simulink model "BaseEngineController_A02"  (Simulink 7.7)
settings: Solver=FixedStepDiscrete  StartTime=0.0  StopTime=10.0  RelTol=1e-3
PreLoadFcn:
  | try, eval([bdroot '_setup']); catch, eval('model_setup', ''); end
InitFcn:
  | try, eval([bdroot '_setup']); catch, eval('model_setup', ''); end
undefined symbols (not defined in the file or in its folder): ECU555, High, motohawk_build_version, motohawk_get_faults, motohawk_trunc_name, motohawk_vardec_config_group, motohawk_vardec_path, True
libraries: MotoHawk_lib x1145, AutoDoc_lib x786, motohawk_control_primitives_lib x114, motohawk_engine_lib x19, motohawk_annotations_lib x12, simulink x11, simulink_extras x1
(part 18 of 23)

== system BaseEngineController_A02/Foreground/Control/Controller/Run/AirFlow Manager/Power Limit Controller ==
  ETC Setpoint : Inport
  Sensors : Inport  Port=2
  Add : Sum
  Add1 : Sum  Inputs=+-
  Bus Selector1 : BusSelector  OutputSignals=TorqueCalcs.Power
  Compare To Zero : simulink/Logic and Bit Operations/Compare To Zero  SystemSampleTime=-1  relop===  LogicOutDataTypeMode=boolean  ZeroCross=off
  Constant1 : Constant  Value=100
  Constant2 : Constant  Value=100
  Documentation_Calibrations_Probes_Block : AutoDoc_lib/Documentation_Calibrations_Probes_Block
  Documentation_Left_Border : AutoDoc_lib/Documentation_Left_Border  layout=Portrait
  Documentation_Right_Border : AutoDoc_lib/Documentation_Right_Border  layout=Portrait
  Documentation_Title_Block : AutoDoc_lib/Documentation_Title_Block
  MinMax : MinMax  Inputs=2
  Power Limiter Setpoint Manager : Stateflow chart  TreatAsAtomicUnit=on
  Unit Delay : UnitDelay  SampleTime=-1
  Universal PID (Tunable & Scheduled) : Subsystem  AncestorBlock=motohawk_control_primitives_lib/Universal PID (Tunable & Scheduled)  mask(Universal PID (Tunable)){nam='PowerLimt', prefix='PowerLimt_', DCOffset=0, PGain=0, IGain=0, DGain=0, ErrorGain=-1, ITermLwrLim=-100, ITermUprLim=0, OutLwrLim=-100, OutUprLim=0, samp_time=-1, units='%', group='Diagnostics & Protection | Power Limit | Power Limit PID Config'}
      mask prompts: nam "Name"; DCOffset "DC Offset"; ErrorGain "Error Pre-scaler Gain"; ITermLwrLim "I-Term Lower Limit"; ITermUprLim "I-Term Upper Limit"; OutLwrLim "PID Output Lower Limit"; OutUprLim "PID Output Upper Limit"; samp_time "Sample Time"; group "MotoTune Group String"
      mask help:
      | This block wraps a 'Universal PID' block to provide gain scheduling and calibrations and probes for most parameters.
      |
      | If 'Sample Time' is -1, then extra code and RAM will be generated to dynamically calculate dt.
      mask init:
      | grp = [group ' | ' nam];
      mask Display:
      | patch([0 0 1 1], [0 0.075 0.075 0], [230/255 0/255 0/255])
      | patch([0 0 1 1], [0.025 0.05 0.05 0.025], [150/255 0/255 0/255])
      | patch([0 0 1 1], [0 0.025 0.025 0], [0/255 0/255 0/255])
      | 
      | disp([nam '\n(PID w/ Gain Schedules)']);
  motohawk_calibration : MotoHawk_lib/Calibration & Probing Blocks/motohawk_calibration  nam='PowerLimit1'  val=60  storage=Calibration  show_more=on  name_source=Use Parameter  data_type=Inherit from 'Default Value'  read_level=1  write_level=1  ovr_cals=on  view_as=Number  enum_struct={'Disabled', 'Enabled'}  vector_style=Wide Row  use_help_units=on  help='Power Limit setpoint corresponding to Level 1.'  units='kW'  use_header_enum=off  row_header_enum={}  col_header_enum={}  use_min_max=off  min_val=-inf  max_val=inf  use_precision=off  precision=''  gain=1  offset=0  exp=1  use_mototune_group=on  mototune_group='Diagnostics & Protection | Power Limit | Power Limit Level Config'
  motohawk_calibration1 : MotoHawk_lib/Calibration & Probing Blocks/motohawk_calibration  nam='PowerLimit2'  val=40  storage=Calibration  show_more=on  name_source=Use Parameter  data_type=Inherit from 'Default Value'  read_level=1  write_level=1  ovr_cals=on  view_as=Number  enum_struct={'Disabled', 'Enabled'}  vector_style=Wide Row  use_help_units=on  help='Power Limit setpoint corresponding to Level 2.'  units='kW'  use_header_enum=off  row_header_enum={}  col_header_enum={}  use_min_max=off  min_val=-inf  max_val=inf  use_precision=off  precision=''  gain=1  offset=0  exp=1  use_mototune_group=on  mototune_group='Diagnostics & Protection | Power Limit | Power Limit Level Config'
  motohawk_calibration2 : MotoHawk_lib/Calibration & Probing Blocks/motohawk_calibration  nam='PowerLimit3'  val=30  storage=Calibration  show_more=on  name_source=Use Parameter  data_type=Inherit from 'Default Value'  read_level=1  write_level=1  ovr_cals=on  view_as=Number  enum_struct={'Disabled', 'Enabled'}  vector_style=Wide Row  use_help_units=on  help='Power Limit setpoint corresponding to Level 3.'  units='kW'  use_header_enum=off  row_header_enum={}  col_header_enum={}  use_min_max=off  min_val=-inf  max_val=inf  use_precision=off  precision=''  gain=1  offset=0  exp=1  use_mototune_group=on  mototune_group='Diagnostics & Protection | Power Limit | Power Limit Level Config'
  motohawk_calibration3 : MotoHawk_lib/Calibration & Probing Blocks/motohawk_calibration  nam='PowerLimit4'  val=20  storage=Calibration  show_more=on  name_source=Use Parameter  data_type=Inherit from 'Default Value'  read_level=1  write_level=1  ovr_cals=on  view_as=Number  enum_struct={'Disabled', 'Enabled'}  vector_style=Wide Row  use_help_units=on  help='Power Limit setpoint corresponding to Level 4.'  units='kW'  use_header_enum=off  row_header_enum={}  col_header_enum={}  use_min_max=off  min_val=-inf  max_val=inf  use_precision=off  precision=''  gain=1  offset=0  exp=1  use_mototune_group=on  mototune_group='Diagnostics & Protection | Power Limit | Power Limit Level Config'
  motohawk_calibration4 : MotoHawk_lib/Calibration & Probing Blocks/motohawk_calibration  nam='EngMaxPower'  val=60  storage=Calibration  show_more=on  name_source=Use Parameter  data_type=Inherit from 'Default Value'  read_level=1  write_level=1  ovr_cals=on  view_as=Number  enum_struct={'Disabled', 'Enabled'}  vector_style=Wide Row  use_help_units=on  help='Maximum power level of engine.'  units='kW'  use_header_enum=off  row_header_enum={}  col_header_enum={}  use_min_max=off  min_val=-inf  max_val=inf  use_precision=off  precision=''  gain=1  offset=0  exp=1  use_mototune_group=on  mototune_group='Diagnostics & Protection | Power Limit | Power Limit Level Config'
  motohawk_fault_action : MotoHawk_lib/Fault Management Blocks/motohawk_fault_action  nam='Level1PowerLimit'  output_status=on  eval_nam=Level1PowerLimit
  motohawk_fault_action1 : MotoHawk_lib/Fault Management Blocks/motohawk_fault_action  nam='Level2PowerLimit'  output_status=on  eval_nam=Level2PowerLimit
  motohawk_fault_action2 : MotoHawk_lib/Fault Management Blocks/motohawk_fault_action  nam='Level3PowerLimit'  output_status=on  eval_nam=Level3PowerLimit
  motohawk_fault_action3 : MotoHawk_lib/Fault Management Blocks/motohawk_fault_action  nam='Level4PowerLimit'  output_status=on  eval_nam=Level4PowerLimit
  motohawk_probe : MotoHawk_lib/Calibration & Probing Blocks/motohawk_probe  nam='PowerLimitETCSetpoint'  show_more=on  name_source=Use Parameter  read_level=1  view_as=Number  enum_struct={'Disabled', 'Enabled'}  vector_style=Wide Row  use_help_units=off  help=''  units=''  use_header_enum=off  row_header_enum={}  col_header_enum={}  use_precision=off  precision=''  gain=1  offset=0  exp=1  use_mototune_group=on  mototune_group='Diagnostics & Protection | Power Limit'
  motohawk_probe1 : MotoHawk_lib/Calibration & Probing Blocks/motohawk_probe  nam='PowerLimitSetpoint'  show_more=on  name_source=Use Parameter  read_level=1  view_as=Number  enum_struct={'Disabled', 'Enabled'}  vector_style=Wide Row  use_help_units=off  help=''  units=''  use_header_enum=off  row_header_enum={}  col_header_enum={}  use_precision=off  precision=''  gain=1  offset=0  exp=1  use_mototune_group=on  mototune_group='Diagnostics & Protection | Power Limit'
  motohawk_probe2 : MotoHawk_lib/Calibration & Probing Blocks/motohawk_probe  nam='PowerLimitState'  show_more=on  name_source=Use Parameter  read_level=1  view_as=Number  enum_struct={'Disabled', 'Enabled'}  vector_style=Wide Row  use_help_units=off  help=''  units=''  use_header_enum=off  row_header_enum={}  col_header_enum={}  use_precision=off  precision=''  gain=1  offset=0  exp=1  use_mototune_group=on  mototune_group='Diagnostics & Protection | Power Limit'
  motohawk_probe3 : MotoHawk_lib/Calibration & Probing Blocks/motohawk_probe  nam='PowerLimitActual'  show_more=on  name_source=Use Parameter  read_level=1  view_as=Number  enum_struct={'Disabled', 'Enabled'}  vector_style=Wide Row  use_help_units=off  help=''  units=''  use_header_enum=off  row_header_enum={}  col_header_enum={}  use_precision=off  precision=''  gain=1  offset=0  exp=1  use_mototune_group=on  mototune_group='Diagnostics & Protection | Power Limit'
  PowerLimitETC : Outport
  wires:
  Constant2/1 -> Add1/2
  Compare To Zero/1 -> Universal PID (Tunable & Scheduled)/2(Reset All)
  Unit Delay/1 -> Power Limiter Setpoint Manager/11(PL_ETC)
  Power Limiter Setpoint Manager/1(State) -> Compare To Zero/1, motohawk_probe2/1
  motohawk_fault_action3/1 -> Power Limiter Setpoint Manager/10(Level4)
  motohawk_fault_action2/1 -> Power Limiter Setpoint Manager/9(Level3)
  motohawk_fault_action1/1 -> Power Limiter Setpoint Manager/8(Level2)
  motohawk_fault_action/1 -> Power Limiter Setpoint Manager/7(Level1)
  Power Limiter Setpoint Manager/2(Setpoint) -> motohawk_probe1/1, Universal PID (Tunable & Scheduled)/4(SetPoint)
  motohawk_calibration3/1 -> Power Limiter Setpoint Manager/6(Level4Setpoint)
  motohawk_calibration2/1 -> Power Limiter Setpoint Manager/5(Level3Setpoint)
  motohawk_calibration1/1 -> Power Limiter Setpoint Manager/4(Level2Setpoint)
  motohawk_calibration/1 -> Power Limiter Setpoint Manager/3(Level1Setpoint)
  motohawk_calibration4/1 -> Power Limiter Setpoint Manager/2(MaxEngPower)
  Bus Selector1/1 -> Universal PID (Tunable & Scheduled)/3(Actual), motohawk_probe3/1  "<Power>"
  Sensors/1 -> Bus Selector1/1
  Constant1/1 -> Add/1
  Add/1 -> Unit Delay/1, motohawk_probe/1, MinMax/2
  Universal PID (Tunable & Scheduled)/1(PIDOut) -> Add/2
  ETC Setpoint/1 -> Add1/1, Power Limiter Setpoint Manager/1(ETC), MinMax/1
  Add1/1 -> Universal PID (Tunable & Scheduled)/1(ITerm IC)
  MinMax/1 -> PowerLimitETC/1

== system BaseEngineController_A02/Foreground/Control/Controller/Run/AirFlow Manager/ShutDownTasksComplete Reset ==
  Enable : EnablePort
  Constant : Constant  Value=boolean(1)
  Documentation_Calibrations_Probes_Block : AutoDoc_lib/Documentation_Calibrations_Probes_Block
  Documentation_Left_Border : AutoDoc_lib/Documentation_Left_Border  layout=Portrait
  Documentation_Right_Border : AutoDoc_lib/Documentation_Right_Border  layout=Portrait
  Documentation_Title_Block : AutoDoc_lib/Documentation_Title_Block
  motohawk_data_write : MotoHawk_lib/Data Storage Blocks/motohawk_data_write  source=Lookup By Name  structure=Scalar  operation=Write entire data structure at once  sz_vec=-1  sz_row=3  sz_col=2  typ=boolean  nam='ShutDownTasksComplete'  checkNull=off  write_read_only=off  struct_inst_name='Struct1'  struct_name='MyStruct'
  wires:
  Constant/1 -> motohawk_data_write/1

== system BaseEngineController_A02/Foreground/Control/Controller/Run/Fuel System Manager/Fuel Calculation ==
  Sensors : Inport
  O2Info : Inport  Port=2
  "Apply Multipliers for O2, Knock Control, and Enrichment1" : Product  SaturateOnIntegerOverflow=on  InputSameDT=on  OutDataTypeStr=Inherit: Same as first input
  Bus Selector : BusSelector  OutputSignals=O2FuelMult
  Bus Selector1 : BusSelector  OutputSignals=DesiredLambda.DesEquivRatio
  Calculate Fuel Flow : Subsystem
  Cals_and_Probes_Page : AutoDoc_lib/Cals_and_Probes_Page  SystemSampleTime=-1
  Data Type Conversion : DataTypeConversion  SaturateOnIntegerOverflow=on  RndMeth=Zero
  Documentation_Calibrations_Probes_Block : AutoDoc_lib/Documentation_Calibrations_Probes_Block
  Documentation_Left_Border : AutoDoc_lib/Documentation_Left_Border  layout=Portrait
  Documentation_Right_Border : AutoDoc_lib/Documentation_Right_Border  layout=Portrait
  Documentation_Title_Block : AutoDoc_lib/Documentation_Title_Block
  Number of Combustion Events Per Revolution : MotoHawk_lib/Data Storage Blocks/motohawk_data_read  source=Lookup By Name  structure=Scalar  operation=Read scalar from location by index  sz_vec=-1  sz_row=-1  sz_col=-1  typ=uint8  nam='CombEventsPerRev'  checkNull=on  struct_inst_name='Struct1'  struct_nam='MyStruct'
  Per-Cylinder Mass Flow Rate : Product  Inputs=*/  SaturateOnIntegerOverflow=on  InputSameDT=on  OutDataTypeStr=Inherit: Same as first input
  motohawk_probe : MotoHawk_lib/Calibration & Probing Blocks/motohawk_probe  nam='GlobalBaseFuelRate'  show_more=on  name_source=Use Parameter  read_level=1  view_as=Number  enum_struct={'Disabled', 'Enabled'}  vector_style=Wide Row  use_help_units=on  help='Liquid fuel rate based on airflow and target equivalence ratio'  units='g/s'  use_header_enum=off  row_header_enum={}  col_header_enum={}  use_precision=off  precision=''  gain=1  offset=0  exp=1  use_mototune_group=on  mototune_group=motohawk_vardec_path(gcb)
  motohawk_probe1 : MotoHawk_lib/Calibration & Probing Blocks/motohawk_probe  nam='PerCylBaseFuelRate'  show_more=on  name_source=Use Parameter  read_level=1  view_as=Number  enum_struct={'Disabled', 'Enabled'}  vector_style=Wide Row  use_help_units=on  help='Liquid fuel mass flow rate based on airflow and target equivalence ratio per cylinder.'  units='g/s'  use_header_enum=off  row_header_enum={}  col_header_enum={}  use_precision=off  precision=''  gain=1  offset=0  exp=1  use_mototune_group=on  mototune_group=motohawk_vardec_path(gcb)
  motohawk_probe2 : MotoHawk_lib/Calibration & Probing Blocks/motohawk_probe  nam='AdjustedPerCylFuelRate'  show_more=on  name_source=Use Parameter  read_level=1  view_as=Number  enum_struct={'Disabled', 'Enabled'}  vector_style=Wide Row  use_help_units=on  help='Desired fuel per cylinder after application of individual cylinder offsets and multipliers.'  units='g/s'  use_header_enum=off  row_header_enum={}  col_header_enum={}  use_precision=off  precision=''  gain=1  offset=0  exp=1  use_mototune_group=on  mototune_group=motohawk_vardec_path(gcb)
  Fuel : Outport
  wires:
  "Apply Multipliers for O2, Knock Control, and Enrichment1"/1 -> Fuel/1, motohawk_probe2/1
  O2Info/1 -> Bus Selector1/1, Bus Selector/1
  Data Type Conversion/1 -> Per-Cylinder Mass Flow Rate/2
  Number of Combustion Events Per Revolution/1 -> Data Type Conversion/1
  Calculate Fuel Flow/1(BaseFuelRate [g/s]) -> Per-Cylinder Mass Flow Rate/1, motohawk_probe/1
  Per-Cylinder Mass Flow Rate/1 -> motohawk_probe1/1, "Apply Multipliers for O2, Knock Control, and Enrichment1"/1
  Sensors/1 -> Calculate Fuel Flow/2(Sensors)
  Bus Selector1/1 -> Calculate Fuel Flow/1(DesEquivRatio)  "<DesEquivRatio>"
  Bus Selector/1 -> "Apply Multipliers for O2, Knock Control, and Enrichment1"/2  "<O2FuelMult>"

== system BaseEngineController_A02/Foreground/Control/Controller/Run/Fuel System Manager/Injector Manager ==
  Fuel : Inport
  Sensors : Inport  Port=2
  Bus Selector : BusSelector  OutputSignals=VirtualIndexes.IndTorqueNom09Idx
  Bus Selector1 : BusSelector  OutputSignals=RawIndexes.RPM09Idx
  Bus Selector2 : BusSelector  OutputSignals=Raw.RPM
  Bus Selector3 : BusSelector  OutputSignals=AirCalcs.MAFPort,Raw.LinearSensors.MAP_Angular,Raw.NonLinearSensors.ECT,Raw.NonLinearSensors.IAT
  Bus Selector4 : BusSelector  OutputSignals=RawProcessed.TPS,AirCalcs.MAFPort,RawIndexes.ECT05Idx
  Constant1 : Constant  OutDataTypeStr=Inherit: Inherit via back propagation
  Delta TPS Transient Fueling : Subsystem  TreatAsAtomicUnit=on
      mask Display:
      | patch([0 0 1 1], [0 0.075 0.075 0], [230/255 0/255 0/255])
      | patch([0 0 1 1], [0.025 0.05 0.05 0.025], [150/255 0/255 0/255])
      | patch([0 0 1 1], [0 0.025 0.025 0], [0/255 0/255 0/255])
      | 
      | disp('TRANSIENT\nFUELING\nMULTIPLIER')
  Divide : Product  Inputs=**
  Documentation_Calibrations_Probes_Block : AutoDoc_lib/Documentation_Calibrations_Probes_Block
  Documentation_Left_Border : AutoDoc_lib/Documentation_Left_Border  layout=Portrait
  Documentation_Right_Border : AutoDoc_lib/Documentation_Right_Border  layout=Portrait
  Documentation_Title_Block : AutoDoc_lib/Documentation_Title_Block
  Make-Up Pulse End of Injection Timing1 : MotoHawk_lib/Lookup Tables/motohawk_interpolation_2d  nam='MakeUpEOI'  table_data=ones(9)*200  show_more=on  data_type=Inherit from 'Table Data'  storage=NonAdaptive  read_level=1  write_level=1  ovr_cals=on  use_help=on  help='End of injection timing for transient makeup fuel pulse'  units='degBTDC'  use_min_max=on  min_val=-1000  max_val=1000  use_precision=on  precision=''  gain=1  offset=0  exp=1  use_links=off  row_prelookup_block=''  col_prelookup_block=''  output_name=''  use_mototune_group=on  mototune_group=motohawk_vardec_path(gcb)
  Mass Flow to Charge Mass Transform : motohawk_engine_lib/Mass Flow to Charge Mass Transform  SystemSampleTime=-1
  Primary Pulse Injection Timing : MotoHawk_lib/Lookup Tables/motohawk_interpolation_2d  nam='PrimarySOI'  table_data=ones(9)*600  show_more=on  data_type=Inherit from 'Table Data'  storage=NonAdaptive  read_level=1  write_level=1  ovr_cals=on  use_help=on  help='Injection timing for primary fuel pulse - SOI or EOI depending on Inj Config'  units='degBTDC'  use_min_max=on  min_val=-1000  max_val=1000  use_precision=on  precision=''  gain=1  offset=0  exp=1  use_links=off  row_prelookup_block=''  col_prelookup_block=''  output_name=''  use_mototune_group=on  mototune_group=motohawk_vardec_path(gcb)
  Tau-X Transient Fueling : Subsystem  AncestorBlock=motohawk_engine_lib/Model Fuel Puddle Mass  mask{group_cal='Engine Control | Run | Fuel | Transient Fuel', group_dis='Engine Control | Run | Fuel | Transient Fuel'}
      mask prompts: group_cal "MotoTune Calibration Group String"; group_dis "MotoTune Display Group String"
      mask Display:
      | patch([0 0 1 1], 0.5*[0 0.075 0.075 0], [230/255 0/255 0/255])
      | patch([0 0 1 1], 0.5*[0.025 0.05 0.05 0.025], [150/255 0/255 0/255])
      | patch([0 0 1 1], 0.5*[0 0.025 0.025 0], [0/255 0/255 0/255])
      | 
      | disp('TAU-X\nTRANSIENT\nFUELING');
  motohawk_calibration1 : MotoHawk_lib/Calibration & Probing Blocks/motohawk_calibration  nam='DeltaTPSFuelingEnable'  val=1  storage=Calibration  show_more=on  name_source=Use Parameter  data_type=boolean  read_level=1  write_level=1  ovr_cals=on  view_as=Enumeration  enum_struct={'Disabled', 'Enabled'}  vector_style=Wide Row  use_help_units=on  help='Enable Delta TPS transient fuel algorithm'  units=''  use_header_enum=off  row_header_enum={}  col_header_enum={}  use_min_max=off  min_val=-inf  max_val=inf  use_precision=off  precision=''  gain=1  offset=0  exp=1  use_mototune_group=on  mototune_group=motohawk_vardec_path(gcb)
  PrimaryInjTiming : Outport
  MakeUpInjTiming : Outport  Port=2
  FPC : Outport  Port=3
  wires:
  Sensors/1 -> Bus Selector3/1, Bus Selector1/1, Bus Selector/1, Bus Selector2/1, Bus Selector4/1
  Bus Selector/1 -> Primary Pulse Injection Timing/1, Make-Up Pulse End of Injection Timing1/1  "<IndTorqueNom09Idx>"
  Bus Selector1/1 -> Primary Pulse Injection Timing/2, Make-Up Pulse End of Injection Timing1/2  "<RPM09Idx>"
  Fuel/1 -> Tau-X Transient Fueling/1(DesFuelRate [g/s])
  Bus Selector2/1 -> Mass Flow to Charge Mass Transform/2  "<RPM>"
  Mass Flow to Charge Mass Transform/1 -> FPC/1
  Constant1/1 -> Mass Flow to Charge Mass Transform/3
  Bus Selector4/1 -> Delta TPS Transient Fueling/1(TPS)  "<TPS>"
  Bus Selector4/2 -> Delta TPS Transient Fueling/2(MAF)  "<MAFPort>"
  Bus Selector4/3 -> Delta TPS Transient Fueling/3(ECTIdx)  "<ECT05Idx>"
  Tau-X Transient Fueling/1(FuelRate [g/s]) -> Divide/2
  Delta TPS Transient Fueling/1(DeltaTPSMult) -> Divide/1
  Divide/1 -> Mass Flow to Charge Mass Transform/1
  motohawk_calibration1/1 -> Delta TPS Transient Fueling/Enable
  Bus Selector3/1 -> Tau-X Transient Fueling/2(MAF [g/s])  "<MAFPort>"
  Bus Selector3/2 -> Tau-X Transient Fueling/3(MAP [kPa])  "<MAP_Angular>"
  Bus Selector3/3 -> Tau-X Transient Fueling/4(ECT [degC])  "<ECT>"
  Bus Selector3/4 -> Tau-X Transient Fueling/5(IAT [degC])  "<IAT>"
  Primary Pulse Injection Timing/1 -> PrimaryInjTiming/1
  Make-Up Pulse End of Injection Timing1/1 -> MakeUpInjTiming/1  "EOI"

== system BaseEngineController_A02/Foreground/Control/Controller/Run/Fuel System Manager/O2 Fuel Adapt ==
  O2 Info : Inport
  Sensors : Inport  Port=2
  WarmUp : Inport  Port=3
  Abs : Abs  SaturateOnIntegerOverflow=on
  Adapt FuelFlowComp Map : Subsystem  TreatAsAtomicUnit=on
  Bus Selector1 : BusSelector  OutputSignals=O2Error.O2ITerm
  Bus Selector2 : BusSelector  OutputSignals=O2ControlEnable.BankAEnable
  Bus Selector3 : BusSelector  OutputSignals=VirtualIndexes.IndTorqueNom09Idx,RawIndexes.RPM09Idx
  Bus Selector5 : BusSelector  OutputSignals=O2Error.ITermIdx
  Cals_and_Probes_Page : AutoDoc_lib/Cals_and_Probes_Page  SystemSampleTime=-1
  Divide : Product  Inputs=**
  Documentation_Calibrations_Probes_Block : AutoDoc_lib/Documentation_Calibrations_Probes_Block
  Documentation_Left_Border : AutoDoc_lib/Documentation_Left_Border  layout=Portrait
  Documentation_Right_Border : AutoDoc_lib/Documentation_Right_Border  layout=Portrait
  Documentation_Title_Block : AutoDoc_lib/Documentation_Title_Block
  Logical Operator2 : Logic  Inputs=5
  O2 Control Enable Delay : Subsystem  TreatAsAtomicUnit=on
  Product0 : Product  SaturateOnIntegerOverflow=on  InputSameDT=on  OutDataTypeStr=Inherit: Same as first input
  Relational Operator : RelationalOperator  Operator=<=  InputSameDT=on
  Saturation (Tunable) : Subsystem  AncestorBlock=motohawk_control_primitives_lib/Saturation (Tunable)  mask(Saturation (Tunable)){prefix='FuelAdaptError', UprLim=1, LwrLim=-1, units='', grp='Engine Control | Run | Fuel | Fuel Adapt | Adapt Config'}
      mask prompts: UprLim "Upper Limit"; LwrLim "Lower Limit"; grp "MotoTune Group String"
      mask help: Saturation block with calibratable min/max values.
      mask Display:
      | patch([0 0 1 1], [0 0.075 0.075 0], [230/255 0/255 0/255])
      | patch([0 0 1 1], [0.025 0.05 0.05 0.025], [150/255 0/255 0/255])
      | patch([0 0 1 1], [0 0.025 0.025 0], [0/255 0/255 0/255])
      | 
      | plot([0 .2 .8 1], [.1 .1 1 1])
  WarmUp Disable : Subsystem  TreatAsAtomicUnit=on
  motohawk_calibration : MotoHawk_lib/Calibration & Probing Blocks/motohawk_calibration  nam='AdaptEnable'  val=true  storage=Display  show_more=on  name_source=Use Parameter  data_type=Inherit from 'Default Value'  read_level=1  write_level=1  ovr_cals=on  view_as=Enumeration  enum_struct={'Disabled', 'Enabled'}  vector_style=Wide Row  use_help_units=on  help='Determines whether the FuelFlowComp map will be adapted using the MAF sensor'  units='bool'  use_header_enum=off  row_header_enum={}  col_header_enum={}  use_min_max=off  min_val=-inf  max_val=inf  use_precision=off  precision=''  gain=1  offset=0  exp=1  use_mototune_group=on  mototune_group=motohawk_vardec_path(gcb)
  motohawk_calibration1 : MotoHawk_lib/Calibration & Probing Blocks/motohawk_calibration  nam='FuelAdaptDelta'  val=0.5  storage=Calibration  show_more=on  name_source=Use Parameter  data_type=Inherit from 'Default Value'  read_level=1  write_level=1  ovr_cals=on  view_as=Number  enum_struct={'Disabled', 'Enabled'}  vector_style=Wide Row  use_help_units=on  help='O2_Error must be less than this delta to adapt the FuelFlowComp map'  units='mult'  use_header_enum=off  row_header_enum={}  col_header_enum={}  use_min_max=on  min_val=0  max_val=2  use_precision=off  precision=''  gain=1  offset=0  exp=1  use_mototune_group=on  mototune_group=motohawk_vardec_path(gcb)
  motohawk_calibration2 : MotoHawk_lib/Calibration & Probing Blocks/motohawk_calibration  nam='FuelAdaptEnable'  val=false  storage=Calibration  show_more=on  name_source=Use Parameter  data_type=Inherit from 'Default Value'  read_level=1  write_level=1  ovr_cals=on  view_as=Enumeration  enum_struct={'Disabled', 'Enabled'}  vector_style=Wide Row  use_help_units=on  help='Determines whether the FuelFlowComp map will be adapted using the MAF sensor'  units='bool'  use_header_enum=off  row_header_enum={}  col_header_enum={}  use_min_max=off  min_val=-inf  max_val=inf  use_precision=off  precision=''  gain=1  offset=0  exp=1  use_mototune_group=on  mototune_group=motohawk_vardec_path(gcb)
  motohawk_interpolation_1d1 : MotoHawk_lib/Lookup Tables/motohawk_interpolation_1d  nam='FuelAdaptGain'  table_data=ones(1,9)  show_more=on  data_type=Inherit from 'Table Data'  storage=NonAdaptive  read_level=1  write_level=1  ovr_cals=on  use_help=on  help="'Gain for updating FuelFlowComp map.  This fraction of the error is added to the map every 5ms.'"  units=''  use_min_max=off  min_val=-inf  max_val=inf  use_precision=on  precision='1.4'  gain=1  offset=0  exp=1  use_links=off  prelookup_block=''  output_name=''  use_mototune_group=on  mototune_group=motohawk_vardec_path(gcb)
  motohawk_interpolation_2d1 : MotoHawk_lib/Lookup Tables/motohawk_interpolation_2d  nam='FuelFlowComp'  table_data=ones(9)  show_more=on  data_type=Inherit from 'Table Data'  storage=NonAdaptive  read_level=1  write_level=1  ovr_cals=on  use_help=on  help='Fuel Flow Compensation Multiplier - gain to adjust modelling errors in mechanical fuel system.'  units='mult'  use_min_max=on  min_val=-inf  max_val=inf  use_precision=on  precision=''  gain=1  offset=0  exp=1  use_links=off  row_prelookup_block=''  col_prelookup_block=''  output_name=''  use_mototune_group=on  mototune_group=motohawk_vardec_path(gcb)
  motohawk_interpolation_2d2 : MotoHawk_lib/Lookup Tables/motohawk_interpolation_2d  nam='FuelFlowCompAdapt'  table_data=ones(9)  show_more=on  data_type=Inherit from 'Table Data'  storage=Adaptive - NonVolatile  read_level=1  write_level=1  ovr_cals=on  use_help=on  help='Adapt map based on O2 ITerm that is multiplied into FuelFlowComp.'  units='mult'  use_min_max=on  min_val=-inf  max_val=inf  use_precision=on  precision=''  gain=1  offset=0  exp=1  use_links=off  row_prelookup_block=''  col_prelookup_block=''  output_name=''  use_mototune_group=on  mototune_group=motohawk_vardec_path(gcb)
  motohawk_probe3 : MotoHawk_lib/Calibration & Probing Blocks/motohawk_probe  nam='FuelAdaptEnabled'  show_more=on  name_source=Use Parameter  read_level=1  view_as=Enumeration  enum_struct={'Not Enabled', 'Enabled'}  vector_style=Wide Row  use_help_units=on  help='Indicates whether FuelFlowComp map adapting is enabled'  units=''  use_header_enum=off  row_header_enum={}  col_header_enum={}  use_precision=off  precision=''  gain=1  offset=0  exp=1  use_mototune_group=on  mototune_group=motohawk_vardec_path(gcb)
  motohawk_probe7 : MotoHawk_lib/Calibration & Probing Blocks/motohawk_probe  nam='FuelAdapt_Error'  show_more=on  name_source=Use Parameter  read_level=1  view_as=Number  enum_struct={'Disabled', 'Enabled'}  vector_style=Wide Row  use_help_units=on  help='Error in fuel flow at the current operating point, as compared to O2 sensor'  units=''  use_header_enum=off  row_header_enum={}  col_header_enum={}  use_precision=off  precision=''  gain=1  offset=0  exp=1  use_mototune_group=on  mototune_group=motohawk_vardec_path(gcb)
  "Adapt Fuel Mult " : Outport
  wires:
  Abs/1 -> Relational Operator/1
  motohawk_calibration1/1 -> Relational Operator/2
  motohawk_interpolation_1d1/1 -> Product0/2
  Product0/1 -> Saturation (Tunable)/1(In)
  Sensors/1 -> Bus Selector3/1
  O2 Info/1 -> Bus Selector1/1, Bus Selector5/1, Bus Selector2/1
  Bus Selector1/1 -> Product0/1, Abs/1  "<O2ITerm>"
  Bus Selector2/1 -> O2 Control Enable Delay/1(State_In)  "<BankAEnable>"
  Bus Selector5/1 -> motohawk_interpolation_1d1/1  "<ITermIdx>"
  Relational Operator/1 -> Logical Operator2/5
  WarmUp Disable/1(State_Out) -> Logical Operator2/3
  WarmUp/1 -> WarmUp Disable/1(Signal_In)
  O2 Control Enable Delay/1(State_Out) -> Logical Operator2/4
  Logical Operator2/1 -> Adapt FuelFlowComp Map/Enable, motohawk_probe3/1
  Saturation (Tunable)/1(Out) -> Adapt FuelFlowComp Map/1(AdaptError), motohawk_probe7/1
  motohawk_calibration/1 -> Logical Operator2/2
  motohawk_calibration2/1 -> Logical Operator2/1
  motohawk_interpolation_2d1/1 -> Divide/1
  Divide/1 -> "Adapt Fuel Mult "/1
  motohawk_interpolation_2d2/1 -> Adapt FuelFlowComp Map/2(Adapt), Divide/2
  Bus Selector3/1 -> motohawk_interpolation_2d2/1, motohawk_interpolation_2d1/1  "<IndTorqueNom09Idx>"
  Bus Selector3/2 -> motohawk_interpolation_2d2/2, motohawk_interpolation_2d1/2  "<RPM09Idx>"

== system BaseEngineController_A02/Foreground/Control/Controller/Run/MaxGovernor/I-Term Preload ==
  TotalETCReq : Inport
  RPMAccelIdx : Inport  Port=2
  Documentation_Calibrations_Probes_Block : AutoDoc_lib/Documentation_Calibrations_Probes_Block
  Documentation_Left_Border : AutoDoc_lib/Documentation_Left_Border  layout=Portrait
  Documentation_Right_Border : AutoDoc_lib/Documentation_Right_Border  layout=Portrait
  Documentation_Title_Block : AutoDoc_lib/Documentation_Title_Block
  Sum : Sum  Inputs=|+-
  motohawk_interpolation_1d4 : MotoHawk_lib/Lookup Tables/motohawk_interpolation_1d  nam='MaxGovPreloadITerm'  table_data=[1 30 50 51.18 52.349998 53.529999 54.709999 55.880001 57.060001 58.240002 59.41 60.59 61.759998 62.939999 67.650002 68.82 70]  show_more=on  data_type=Inherit from 'Table Data'  storage=NonAdaptive  read_level=1  write_level=1  ovr_cals=on  use_help=on  help='Preload for Max Gov PID ITerm based on engine acceleration rate upon entry into max gov.'  units='%'  use_min_max=on  min_val=-inf  max_val=inf  use_precision=on  precision=''  gain=1  offset=0  exp=1  use_links=off  prelookup_block=''  output_name=''  use_mototune_group=on  mototune_group=motohawk_vardec_path(gcb)
  motohawk_probe : MotoHawk_lib/Calibration & Probing Blocks/motohawk_probe  nam='MaxGovIC'  show_more=on  name_source=Use Parameter  read_level=1  view_as=Number  enum_struct={'Disabled', 'Enabled'}  vector_style=Wide Row  use_help_units=off  help=''  units=''  use_header_enum=off  row_header_enum={}  col_header_enum={}  use_precision=off  precision=''  gain=1  offset=0  exp=1  use_mototune_group=on  mototune_group=motohawk_vardec_path(gcb)
  ITerm Preload : Outport
  wires:
  RPMAccelIdx/1 -> motohawk_interpolation_1d4/1
  Sum/1 -> ITerm Preload/1, motohawk_probe/1
  motohawk_interpolation_1d4/1 -> Sum/2
  TotalETCReq/1 -> Sum/1

== system BaseEngineController_A02/Foreground/Control/Controller/Run/MaxGovernor/MaxGovState ==
  note: Move into On State if RPM > EntryCriteria or Target.
      | Move into Trans State if APP falls below TPS, PID controller is frozen during Trans State, but output is based on APP.
      | 	When in AllSpeedGov mode, switch to Trans if AllSpeedGov setpoint is less than MaxGov setpoint minus hyst.
      | Move from Trans State back to On State if RPM > Target.
      | Move from Trans State to Off if RPM < Entry Criteria.
  RPMEntryCriteria : Inport
  TotalETCReq : Inport  Port=2
  Sensors : Inport  Port=3
  TargetRPM : Inport  Port=4
  Bus Selector : BusSelector  OutputSignals=RawProcessed.TPS
  Bus Selector1 : BusSelector  OutputSignals=Raw.RPMInst
  Constant : Constant  Value=boolean(0)
  Data Type Conversion : DataTypeConversion  OutDataTypeStr=int8
  Data Type Conversion1 : DataTypeConversion  OutDataTypeStr=int8
  Data Type Conversion2 : DataTypeConversion  OutDataTypeStr=int8
  Documentation_Calibrations_Probes_Block : AutoDoc_lib/Documentation_Calibrations_Probes_Block
  Documentation_Left_Border : AutoDoc_lib/Documentation_Left_Border  layout=Portrait
  Documentation_Right_Border : AutoDoc_lib/Documentation_Right_Border  layout=Portrait
  Documentation_Title_Block : AutoDoc_lib/Documentation_Title_Block
  Logical Operator : Logic  Operator=NOT  AllPortsSameDT=off  OutDataTypeStr=boolean
  Logical Operator1 : Logic  AllPortsSameDT=off  OutDataTypeStr=boolean
  Logical Operator2 : Logic  Operator=OR  AllPortsSameDT=off  OutDataTypeStr=boolean
  Logical Operator3 : Logic  Operator=OR  AllPortsSameDT=off  OutDataTypeStr=boolean
  MaxGOV Stateflow : Stateflow chart  TreatAsAtomicUnit=on
  Multiport Switch : MultiPortSwitch  DataPortOrder=Zero-based contiguous  Inputs=2  zeroidx=on
  Relational Operator : RelationalOperator  Operator=<=  OutDataTypeStr=boolean
  Relational Operator1 : RelationalOperator  Operator=>  OutDataTypeStr=boolean
  Relational Operator2 : RelationalOperator  Operator=>  OutDataTypeStr=boolean
  Sum : Sum  Inputs=|+-
  Sum1 : Sum  Inputs=++|
  motohawk_calibration1 : MotoHawk_lib/Calibration & Probing Blocks/motohawk_calibration  nam='MaxGovDisable'  val=0  storage=Calibration  show_more=on  name_source=Use Parameter  data_type=boolean  read_level=1  write_level=1  ovr_cals=on  view_as=Enumeration  enum_struct={'Gov Enabled', 'Gov Disabled'}  vector_style=Wide Row  use_help_units=on  help='Max governor manual disable'  units=''  use_header_enum=off  row_header_enum={}  col_header_enum={}  use_min_max=off  min_val=0  max_val=inf  use_precision=off  precision=''  gain=1  offset=0  exp=1  use_mototune_group=on  mototune_group=motohawk_vardec_path(gcb)
  motohawk_calibration5 : MotoHawk_lib/Calibration & Probing Blocks/motohawk_calibration  nam='MaxGovTPSHyst'  val=4  storage=Calibration  show_more=on  name_source=Use Parameter  data_type=Inherit from 'Default Value'  read_level=1  write_level=1  ovr_cals=on  view_as=Number  enum_struct={'Disabled', 'Enabled'}  vector_style=Wide Row  use_help_units=on  help='Hysterisis around max gov TPS entry criteria.'  units='%'  use_header_enum=off  row_header_enum={}  col_header_enum={}  use_min_max=on  min_val=0  max_val=inf  use_precision=on  precision=''  gain=1  offset=0  exp=1  use_mototune_group=on  mototune_group=motohawk_vardec_path(gcb)
  motohawk_data_def : MotoHawk_lib/Data Storage Blocks/motohawk_data_def  nam='MaxGov'  container_nam=''  data=0  typ=double  struct_nam='MyStruct'  storage=Volatile  outputRef=off  use_vardec=on  window=Display  read_level=1  write_level=1  ovr_cals=on  view_as=Number  enum_struct={'Disabled', 'Enabled'}  vector_style=Wide Row  use_help_units=off  help=''  units=''  use_header_enum=off  row_header_enum=''  col_header_enum=''  use_min_max=off  min_val=-inf  max_val=inf  use_precision=off  precision=''  gain=1  offset=0  exp=1  use_mototune_group=off  mototune_group=motohawk_vardec_path(gcb)  eval_name=MyData  data_stores=motohawk_sfun_data_def_data_stores  struct_instances=motohawk_sfun_data_def_struct_instances  vardecs=motohawk_sfun_data_def_vardecs
  motohawk_data_read : MotoHawk_lib/Data Storage Blocks/motohawk_data_read  source=Lookup By Name  structure=Scalar  operation=Read scalar from location by index  sz_vec=4  sz_row=2  sz_col=3  typ=double  nam='MaxGov'  checkNull=on  struct_inst_name='MyData'  struct_nam='MyStruct'
  motohawk_data_write : MotoHawk_lib/Data Storage Blocks/motohawk_data_write  source=Lookup By Name  structure=Scalar  operation=Write entire data structure at once  sz_vec=9  sz_row=2  sz_col=9  typ=double  nam='MaxGov'  checkNull=on  write_read_only=off  struct_inst_name=''  struct_name='MyStruct'
  motohawk_probe : MotoHawk_lib/Calibration & Probing Blocks/motohawk_probe  nam='MaxGovETCMode'  show_more=on  name_source=Use Parameter  read_level=1  view_as=Enumeration  enum_struct={'ETC Req Mode','PID Mode'}  vector_style=Wide Row  use_help_units=on  help=''  units=''  use_header_enum=off  row_header_enum={}  col_header_enum={}  use_precision=on  precision=''  gain=1  offset=0  exp=1  use_mototune_group=on  mototune_group=motohawk_vardec_path(gcb)
  motohawk_probe1 : MotoHawk_lib/Calibration & Probing Blocks/motohawk_probe  nam='MaxGovHold'  show_more=on  name_source=Use Parameter  read_level=1  view_as=Enumeration  enum_struct={'Hold Off','Hold On'}  vector_style=Wide Row  use_help_units=on  help=''  units=''  use_header_enum=off  row_header_enum={}  col_header_enum={}  use_precision=on  precision=''  gain=1  offset=0  exp=1  use_mototune_group=on  mototune_group=motohawk_vardec_path(gcb)
  motohawk_probe2 : MotoHawk_lib/Calibration & Probing Blocks/motohawk_probe  nam='MaxGovReset'  show_more=on  name_source=Use Parameter  read_level=1  view_as=Enumeration  enum_struct={'Reset Off','Reset On'}  vector_style=Wide Row  use_help_units=on  help=''  units=''  use_header_enum=off  row_header_enum={}  col_header_enum={}  use_precision=on  precision=''  gain=1  offset=0  exp=1  use_mototune_group=on  mototune_group=motohawk_vardec_path(gcb)
  motohawk_probe4 : MotoHawk_lib/Calibration & Probing Blocks/motohawk_probe  nam='MaxGovState'  show_more=on  name_source=Use Parameter  read_level=1  view_as=Enumeration  enum_struct={'Disabled', 'Enabled'}  vector_style=Wide Row  use_help_units=off  help=''  units=''  use_header_enum=off  row_header_enum={}  col_header_enum={}  use_precision=off  precision=''  gain=1  offset=0  exp=1  use_mototune_group=on  mototune_group=motohawk_vardec_path(gcb)
  ETCMode : Outport
  PIDReset : Outport  Port=2
  PIDHold : Outport  Port=3
  wires:
  Relational Operator/1 -> MaxGOV Stateflow/2(OnToTrans)
  motohawk_calibration5/1 -> Sum/2, Sum1/1
  Sensors/1 -> Bus Selector/1, Bus Selector1/1
  Bus Selector/1 -> Sum/1, Sum1/2  "<TPS>"
  Sum/1 -> Relational Operator/2
  Sum1/1 -> Relational Operator1/2
  TotalETCReq/1 -> Relational Operator/1, Relational Operator1/1
  RPMEntryCriteria/1 -> Logical Operator1/1, Logical Operator/1, Logical Operator3/1
  Relational Operator1/1 -> Logical Operator1/2
  MaxGOV Stateflow/3(Hold) -> Data Type Conversion1/1
  Logical Operator/1 -> MaxGOV Stateflow/4(TransToOff)
  Bus Selector1/1 -> Relational Operator2/1  "<RPMInst>"
  TargetRPM/1 -> Relational Operator2/2
  Logical Operator2/1 -> MaxGOV Stateflow/3(TransToOn)
  Logical Operator1/1 -> Logical Operator2/1
  Relational Operator2/1 -> Logical Operator2/2, Logical Operator3/2
  MaxGOV Stateflow/2(Reset) -> Data Type Conversion/1
  Logical Operator3/1 -> MaxGOV Stateflow/1(OffToOn)
  motohawk_calibration1/1 -> Multiport Switch/1
  MaxGOV Stateflow/1(ETCMode) -> Data Type Conversion2/1
  Multiport Switch/1 -> ETCMode/1, motohawk_probe4/1, motohawk_probe/1
  Constant/1 -> Multiport Switch/3
  MaxGOV Stateflow/4(StateO) -> motohawk_data_write/1
  motohawk_data_read/1 -> MaxGOV Stateflow/5(State)
  Data Type Conversion/1 -> PIDReset/1, motohawk_probe2/1
  Data Type Conversion1/1 -> PIDHold/1, motohawk_probe1/1
  Data Type Conversion2/1 -> Multiport Switch/2

== system BaseEngineController_A02/Foreground/Control/Controller/Run/MaxGovernor/Ramp RPM ==
  note: 1st-Order Ramp Up
  note: y[k] = a*x[k] + (1-a)*y[k-1]
  note: where a = t/T
  OnIdle : Inport
  TargetRPM : Inport  Port=2
  RPM : Inport  Port=3
  Constant : Constant  OutDataTypeStr=Inherit: Inherit via back propagation
  Documentation_Calibrations_Probes_Block : AutoDoc_lib/Documentation_Calibrations_Probes_Block
  Documentation_Left_Border : AutoDoc_lib/Documentation_Left_Border  layout=Portrait
  Documentation_Right_Border : AutoDoc_lib/Documentation_Right_Border  layout=Portrait
  Documentation_Title_Block : AutoDoc_lib/Documentation_Title_Block
  Don't allow setpoint to ever go above the TargetRPM : MinMax  Inputs=2  SaturateOnIntegerOverflow=on  InputSameDT=on
  Logical Operator : Logic  Operator=NOT
  Preload lowpass filter with current RPM while not on Idle : Switch  Threshold=0.5  ZeroCross=off  SaturateOnIntegerOverflow=on  InputSameDT=on
  Product1 : Product  Inputs=*/  SaturateOnIntegerOverflow=on  InputSameDT=on  OutDataTypeStr=Inherit: Same as first input
  Product2 : Product  SaturateOnIntegerOverflow=on  InputSameDT=on  OutDataTypeStr=Inherit: Same as first input
  Product3 : Product  SaturateOnIntegerOverflow=on  InputSameDT=on  OutDataTypeStr=Inherit: Same as first input
  Sum : Sum  Inputs=-+|  SaturateOnIntegerOverflow=on
  Sum1 : Sum  SaturateOnIntegerOverflow=on
  Unit Delay : UnitDelay  SampleTime=-1
  motohawk_calibration2 : MotoHawk_lib/Calibration & Probing Blocks/motohawk_calibration  nam='MaxGovRPMCtrlEntryFiltC'  val=0.2  storage=Calibration  show_more=on  name_source=Use Parameter  data_type=Inherit from 'Default Value'  read_level=1  write_level=1  ovr_cals=on  view_as=Number  enum_struct={'Disabled', 'Enabled'}  vector_style=Wide Row  use_help_units=on  help='Filter rate at which Max Gov setup is increased to MaxGovBaseRPMSetpoint.'  units='sec'  use_header_enum=off  row_header_enum={}  col_header_enum={}  use_min_max=on  min_val=.005  max_val=inf  use_precision=off  precision=''  gain=1  offset=0  exp=1  use_mototune_group=on  mototune_group=motohawk_vardec_path(gcb)
  motohawk_delta_time : MotoHawk_lib/Extra Development Blocks/motohawk_delta_time  samp_time=-1  init_val=0  mode=Seconds (double)
  motohawk_probe3 : MotoHawk_lib/Calibration & Probing Blocks/motohawk_probe  nam='MaxGovRPMSetpoint'  show_more=on  name_source=Use Parameter  read_level=1  view_as=Number  enum_struct={'Disabled', 'Enabled'}  vector_style=Wide Row  use_help_units=on  help='RPMSetpoint of Max Gov controller.'  units='RPM'  use_header_enum=off  row_header_enum={}  col_header_enum={}  use_precision=on  precision=''  gain=1  offset=0  exp=1  use_mototune_group=on  mototune_group=motohawk_vardec_path(gcb)
  RPMSetPt : Outport
  wires:
  Logical Operator/1 -> Preload lowpass filter with current RPM while not on Idle/2
  Don't allow setpoint to ever go above the TargetRPM/1 -> RPMSetPt/1, Unit Delay/1, motohawk_probe3/1
  Preload lowpass filter with current RPM while not on Idle/1 -> Don't allow setpoint to ever go above the TargetRPM/2
  RPM/1 -> Preload lowpass filter with current RPM while not on Idle/1
  TargetRPM/1 -> Don't allow setpoint to ever go above the TargetRPM/1, Product2/1
  Product1/1 -> Sum/1, Product2/2  "alpha"
  Constant/1 -> Sum/2
  Product2/1 -> Sum1/1
  Product3/1 -> Sum1/2
  Sum/1 -> Product3/1
  Unit Delay/1 -> Product3/2
  motohawk_delta_time/1 -> Product1/1
  motohawk_calibration2/1 -> Product1/2
  Sum1/1 -> Preload lowpass filter with current RPM while not on Idle/3
  OnIdle/1 -> Logical Operator/1

== system BaseEngineController_A02/Foreground/Control/Controller/Run/MaxGovernor/Trigger Pre-load ==
  RPM : Inport
  GearState : Inport  Port=2
  Documentation_Calibrations_Probes_Block : AutoDoc_lib/Documentation_Calibrations_Probes_Block
  Documentation_Left_Border : AutoDoc_lib/Documentation_Left_Border  layout=Portrait
  Documentation_Right_Border : AutoDoc_lib/Documentation_Right_Border  layout=Portrait
  Documentation_Title_Block : AutoDoc_lib/Documentation_Title_Block
  InGear : Subsystem  TreatAsAtomicUnit=on
  InNeutral : Subsystem  TreatAsAtomicUnit=on
  Logical Operator : Logic  Operator=NOT  AllPortsSameDT=off  OutDataTypeStr=boolean
  Merge : Merge
  Merge1 : Merge
  Relational Operator : RelationalOperator  Operator=>  OutDataTypeStr=boolean
  Sum1 : Sum  Inputs=+-|
  Trigger : Outport
  BaseRPMSetpoint : Outport  Port=2
  wires:
  Relational Operator/1 -> Trigger/1
  RPM/1 -> Relational Operator/1
  Sum1/1 -> Relational Operator/2
  InGear/1(BaseSetpoint) -> Merge/1
  InGear/2(Threshold) -> Merge1/1
  InNeutral/1(BaseSetpoint) -> Merge/2
  InNeutral/2(Threshold) -> Merge1/2
  Merge/1 -> Sum1/1, BaseRPMSetpoint/1
  Merge1/1 -> Sum1/2
  GearState/1 -> InGear/Enable, Logical Operator/1
  Logical Operator/1 -> InNeutral/Enable
